simulink model "CDF_VSVPWM_Grid_Parallel"  (R2024b)
settings: Solver=VariableStepAuto  StartTime=0.0  StopTime=0.1  FixedStep=1e-7  MaxStep=1e-5  RelTol=1e-4
InitFcn:
  | Ts=1/10e3;
  | Udc=600;
  | C=4000e-6;
  | m=0.7;
libraries: simulink x24, spsMosfetLib x24, spsSeriesRLCBranchLib x16, spsDiodeLib x12, spsabctoAlphaBetaZeroLib x5, spsThreePhaseVIMeasurementLib x4, spsVoltageMeasurementLib x4, spsAlphaBetaZerotoabcLib x2, spsStairGeneratorLib x2, spsOnDelayLib x2, spsdq0toAlphaBetaZeroLib x2, spsBreakerLib x1
(part 2 of 5)

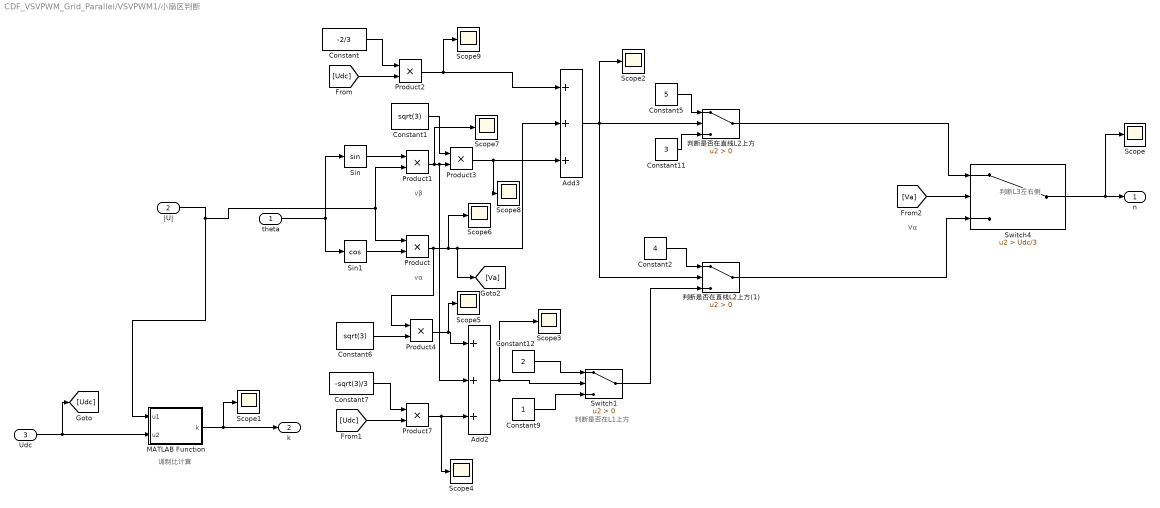
== system CDF_VSVPWM_Grid_Parallel/VSVPWM1/小扇区判断 ==
  note: Vα
  note: 判断L3左右侧
  note: vα
  note: 调制比计算
  note: vβ
  note: 判断是否在L1上方
  theta : Inport
  |U| : Inport  Port=2
  Udc : Inport  Port=3
  "   判断是否在直线L2上方" : Switch  Criteria=u2 > Threshold  SaturateOnIntegerOverflow=off  InputSameDT=off
  "   判断是否在直线L2上方(1)" : Switch  Criteria=u2 > Threshold  SaturateOnIntegerOverflow=off  InputSameDT=off
  Add2 : Sum  Inputs=+++
  Add3 : Sum  Inputs=+++
  Constant : Constant  Value=-2/3
  Constant1 : Constant  Value=sqrt(3)
  Constant11 : Constant  Value=3
  Constant12 : Constant  Value=2
  Constant2 : Constant  Value=4
  Constant5 : Constant  Value=5
  Constant6 : Constant  Value=sqrt(3)
  Constant7 : Constant  Value=-sqrt(3)/3
  Constant9 : Constant
  MATLAB Function : MATLAB Function  TreatAsAtomicUnit=on
  Product{,1..4,7} (6 alike) : Product
  Scope{,1..9} (10 alike) : Scope
  Sin : Trigonometry
  Sin1 : Trigonometry  Operator=cos
  Switch1 : Switch  Criteria=u2 > Threshold  SaturateOnIntegerOverflow=off  InputSameDT=off
  Switch4 : Switch  Criteria=u2 > Threshold  Threshold=Udc/3  SaturateOnIntegerOverflow=off  InputSameDT=off
  n : Outport
  k : Outport  Port=2
  wires:
  Udc/1 -> [Udc], MATLAB Function/2(u2)
  |U|/1 -> MATLAB Function/1(u1), Product1/2, Product/1
  MATLAB Function/1(k) -> Scope1/1, k/1
  theta/1 -> Sin1/1, Sin/1
  Constant/1 -> Product2/1
  [Udc] -> Product2/2
  Constant5/1 -> "   判断是否在直线L2上方"/1
  Switch4/1 -> Scope/1, n/1
  Sin/1 -> Product1/1
  Sin1/1 -> Product/2
  Constant11/1 -> "   判断是否在直线L2上方"/3
  Product1/1 -> Add2/2, Product3/2, Scope7/1
  Constant1/1 -> Product3/1
  Product3/1 -> Scope8/1, Add3/3
  Product/1 -> Scope6/1, [Va], Add3/2, Product4/1
  Product2/1 -> Scope9/1, Add3/1
  Add3/1 -> "   判断是否在直线L2上方(1)"/2, Scope2/1, "   判断是否在直线L2上方"/2
  "   判断是否在直线L2上方"/1 -> Switch4/1
  Constant2/1 -> "   判断是否在直线L2上方(1)"/1
  Constant12/1 -> Switch1/1
  Constant9/1 -> Switch1/3
  Constant6/1 -> Product4/2
  Product4/1 -> Scope5/1, Add2/1
  [Udc] -> Product7/2
  Constant7/1 -> Product7/1
  Product7/1 -> Scope4/1, Add2/3
  Add2/1 -> Scope3/1, Switch1/2
  Switch1/1 -> "   判断是否在直线L2上方(1)"/3
  "   判断是否在直线L2上方(1)"/1 -> Switch4/3
  [Va] -> Switch4/2

== system CDF_VSVPWM_Grid_Parallel/VSVPWM1/生成时间调制波 ==
  note: 调制信号生成
  note: 小扇区
  note: 大扇区
  N : Inport
  n : Inport  Port=2
  T1 : Inport  Port=3
  T2 : Inport  Port=4
  T3 : Inport  Port=5
  T4 : Inport  Port=6
  T5 : Inport  Port=7
  Demux : Demux  Outputs=6
  Gain : Gain  Gain=1/Ts
  Multiport Switch : MultiPortSwitch  Inputs=6  SaturateOnIntegerOverflow=off  InputSameDT=off
  Mux : Mux  Inputs=5
  Scope10 : Scope  NumInputPorts=6
  Scope11 : Scope  NumInputPorts=3
  Scope12 : Scope  NumInputPorts=3
  Scope13 : Scope  NumInputPorts=3
  Scope6 : Scope
  Scope9 : Scope  NumInputPorts=2
  Ⅰ扇区的调制信号计算 : Subsystem
  Ⅱ扇区的调制信号计算 : Subsystem
  Ⅲ扇区的调制信号计算1 : Subsystem
  Ⅳ扇区的调制信号计算 : Subsystem
  Ⅴ扇区的调制信号计算 : Subsystem
  Ⅵ扇区的调制信号计算 : Subsystem
  Tabc12 : Outport
  wires:
  T2/1 -> Mux/2
  T3/1 -> Mux/3
  T1/1 -> Mux/1
  Mux/1 -> Ⅳ扇区的调制信号计算/2(T12345), Ⅵ扇区的调制信号计算/2(T12345), Ⅴ扇区的调制信号计算/2(T12345), Ⅲ扇区的调制信号计算1/2(T12345), Ⅱ扇区的调制信号计算/2(T12345), Ⅰ扇区的调制信号计算/2(T12345)
  n/1 -> Ⅵ扇区的调制信号计算/1(n), Ⅴ扇区的调制信号计算/1(n), Ⅳ扇区的调制信号计算/1(n), Ⅲ扇区的调制信号计算1/1(n), Ⅱ扇区的调制信号计算/1(n), Ⅰ扇区的调制信号计算/1(n)
  Ⅰ扇区的调制信号计算/1(Tabc12) -> Scope12/2, Multiport Switch/2
  Ⅱ扇区的调制信号计算/1(Tabc12) -> Scope11/2, Multiport Switch/3
  N/1 -> Scope13/2, Scope11/1, Scope12/1, Scope9/2, Multiport Switch/1
  Ⅲ扇区的调制信号计算1/1(Tabc12) -> Scope13/1, Multiport Switch/4
  Ⅴ扇区的调制信号计算/1(Tabc12) -> Multiport Switch/6
  Ⅳ扇区的调制信号计算/1(Tabc12) -> Multiport Switch/5
  Ⅵ扇区的调制信号计算/1(Tabc12) -> Scope6/1, Multiport Switch/7
  T4/1 -> Mux/4
  T5/1 -> Mux/5
  ? -> ?
  Gain/1 -> Scope9/1, Tabc12/1
  Multiport Switch/1 -> Scope13/3, Scope11/3, Scope12/3, Demux/1, Gain/1
  Demux/1 -> Scope10/1
  Demux/2 -> Scope10/2
  Demux/3 -> Scope10/3
  Demux/4 -> Scope10/4
  Demux/5 -> Scope10/5
  Demux/6 -> Scope10/6

== system CDF_VSVPWM_Grid_Parallel/VSVPWM1/矢量作用时间计算 ==
  note: 矢量作用时长
  note: 小扇区
  note: 目标矢量角度
  note: 采样时间
  note: 调制比
  n : Inport
  k : Inport  Port=2
  theta : Inport  Port=3
  Ts : Inport  Port=4
  Demux : Demux  Outputs=3
  I1 : Subsystem
  I2 : Subsystem
  I3 : Subsystem
  I4 : Subsystem
  I5 : Subsystem
  Multiport Switch : MultiPortSwitch  Inputs=5  SaturateOnIntegerOverflow=off  InputSameDT=off
  Scope : Scope  NumInputPorts=3
  Ta : Outport
  Tb : Outport  Port=2
  Tc : Outport  Port=3
  wires:
  theta/1 -> I2/3(theta), I3/3(theta), I5/3(theta), I4/3(theta), I1/3(theta)
  Ts/1 -> I2/2(Ts), I3/2(Ts), I5/2(Ts), I4/2(Ts), I1/2(Ts)
  k/1 -> I2/1(k), I3/1(k), I5/1(k), I4/1(k), I1/1(k)
  n/1 -> Multiport Switch/1
  Multiport Switch/1 -> Demux/1
  I1/1(T) -> Multiport Switch/2
  I2/1(T) -> Multiport Switch/3
  I3/1(T) -> Multiport Switch/4
  I4/1(T) -> Multiport Switch/5
  I5/1(T) -> Multiport Switch/6
  Demux/1 -> Scope/1, Ta/1
  Demux/2 -> Scope/2, Tb/1
  Demux/3 -> Scope/3, Tc/1

== system CDF_VSVPWM_Grid_Parallel/VSVPWM/生成时间调制波/Ⅰ扇区的调制信号计算 ==
  n : Inport
  T12345 : Inport  Port=2
  1-1 : Subsystem
  1-2 : Subsystem
  1-3 : Subsystem
  1-4 : Subsystem
  1-5 : Subsystem
  Multiport Switch : MultiPortSwitch  Inputs=5  DiagnosticForDefault=None  SaturateOnIntegerOverflow=off  InputSameDT=off
  Scope : Scope  NumInputPorts=7
  Tabc12 : Outport
  wires:
  n/1 -> Scope/6, Multiport Switch/1
  T12345/1 -> 1-3/1(time), 1-5/1(time), 1-4/1(time), 1-2/1(time), 1-1/1(time)
  1-1/1(Tabc12) -> Scope/1, Multiport Switch/2
  1-2/1(Tabc12) -> Scope/2, Multiport Switch/3
  1-3/1(Tabc12) -> Scope/3, Multiport Switch/4
  1-4/1(Tabc12) -> Scope/4, Multiport Switch/5
  1-5/1(Tabc12) -> Scope/5, Multiport Switch/6
  Multiport Switch/1 -> Scope/7, Tabc12/1

== system CDF_VSVPWM_Grid_Parallel/VSVPWM/生成时间调制波/Ⅱ扇区的调制信号计算 ==
  n : Inport
  T12345 : Inport  Port=2
  2-1 : Subsystem
  2-2 : Subsystem
  2-3 : Subsystem
  2-4 : Subsystem
  2-5 : Subsystem
  Multiport Switch : MultiPortSwitch  Inputs=5  DiagnosticForDefault=None  SaturateOnIntegerOverflow=off  InputSameDT=off
  Scope : Scope  NumInputPorts=7
  Tabc12 : Outport
  wires:
  Multiport Switch/1 -> Scope/7, Tabc12/1
  2-5/1(Tabc12) -> Scope/5, Multiport Switch/6
  2-4/1(Tabc12) -> Scope/4, Multiport Switch/5
  2-3/1(Tabc12) -> Multiport Switch/4, Scope/3
  2-2/1(Tabc12) -> Multiport Switch/3, Scope/2
  2-1/1(Tabc12) -> Scope/1, Multiport Switch/2
  T12345/1 -> 2-2/1(time), 2-1/1(time), 2-3/1(time), 2-5/1(time), 2-4/1(time)
  n/1 -> Multiport Switch/1, Scope/6

== system CDF_VSVPWM_Grid_Parallel/VSVPWM/生成时间调制波/Ⅲ扇区的调制信号计算1 ==
  n : Inport
  T12345 : Inport  Port=2
  1-1 : Subsystem
  1-2 : Subsystem
  1-3 : Subsystem
  1-4 : Subsystem
  1-5 : Subsystem
  Multiport Switch : MultiPortSwitch  Inputs=5  DiagnosticForDefault=None  SaturateOnIntegerOverflow=off  InputSameDT=off
  Scope : Scope  NumInputPorts=7
  Scope1 : Scope
  Tabc12 : Outport
  wires:
  Multiport Switch/1 -> Scope1/1, Scope/7, Tabc12/1
  1-5/1(Tabc12) -> Scope/5, Multiport Switch/6
  1-4/1(Tabc12) -> Scope/4, Multiport Switch/5
  1-3/1(Tabc12) -> Scope/3, Multiport Switch/4
  1-2/1(Tabc12) -> Scope/2, Multiport Switch/3
  1-1/1(Tabc12) -> Scope/1, Multiport Switch/2
  T12345/1 -> 1-1/1(time), 1-2/1(time), 1-4/1(time), 1-5/1(time), 1-3/1(time)
  n/1 -> Multiport Switch/1, Scope/6

== system CDF_VSVPWM_Grid_Parallel/VSVPWM/生成时间调制波/Ⅳ扇区的调制信号计算 ==
  n : Inport
  T12345 : Inport  Port=2
  2-1 : Subsystem
  2-2 : Subsystem
  2-3 : Subsystem
  2-4 : Subsystem
  2-5 : Subsystem
  Multiport Switch : MultiPortSwitch  Inputs=5  DiagnosticForDefault=None  SaturateOnIntegerOverflow=off  InputSameDT=off
  Scope : Scope  NumInputPorts=7
  Scope1 : Scope
  Tabc12 : Outport
  wires:
  n/1 -> Scope/6, Multiport Switch/1
  T12345/1 -> 2-4/1(time), 2-5/1(time), 2-3/1(time), 2-1/1(time), 2-2/1(time)
  2-1/1(Tabc12) -> Scope/1, Multiport Switch/2
  2-2/1(Tabc12) -> Scope/2, Multiport Switch/3
  2-3/1(Tabc12) -> Scope/3, Multiport Switch/4
  2-4/1(Tabc12) -> Scope/4, Multiport Switch/5
  2-5/1(Tabc12) -> Scope/5, Multiport Switch/6
  Multiport Switch/1 -> Scope1/1, Scope/7, Tabc12/1

== system CDF_VSVPWM_Grid_Parallel/VSVPWM/生成时间调制波/Ⅴ扇区的调制信号计算 ==
  n : Inport
  T12345 : Inport  Port=2
  1-1 : Subsystem
  1-2 : Subsystem
  1-3 : Subsystem
  1-4 : Subsystem
  1-5 : Subsystem
  Multiport Switch : MultiPortSwitch  Inputs=5  DiagnosticForDefault=None  SaturateOnIntegerOverflow=off  InputSameDT=off
  Scope : Scope  NumInputPorts=7
  Scope1 : Scope
  Tabc12 : Outport
  wires:
  Multiport Switch/1 -> Scope1/1, Scope/7, Tabc12/1
  1-5/1(Tabc12) -> Scope/5, Multiport Switch/6
  1-4/1(Tabc12) -> Scope/4, Multiport Switch/5
  1-3/1(Tabc12) -> Scope/3, Multiport Switch/4
  1-2/1(Tabc12) -> Multiport Switch/3, Scope/2
  1-1/1(Tabc12) -> Scope/1, Multiport Switch/2
  T12345/1 -> 1-1/1(time), 1-2/1(time), 1-4/1(time), 1-5/1(time), 1-3/1(time)
  n/1 -> Scope/6, Multiport Switch/1

== system CDF_VSVPWM_Grid_Parallel/VSVPWM/生成时间调制波/Ⅵ扇区的调制信号计算 ==
  n : Inport
  T12345 : Inport  Port=2
  2-1 : Subsystem
  2-2 : Subsystem
  2-3 : Subsystem
  2-4 : Subsystem
  2-5 : Subsystem
  Multiport Switch : MultiPortSwitch  Inputs=5  DiagnosticForDefault=None  SaturateOnIntegerOverflow=off  InputSameDT=off
  Tabc12 : Outport
  wires:
  n/1 -> Multiport Switch/1
  T12345/1 -> 2-4/1(time), 2-5/1(time), 2-3/1(time), 2-1/1(time), 2-2/1(time)
  2-1/1(Tabc12) -> Multiport Switch/2
  2-2/1(Tabc12) -> Multiport Switch/3
  2-3/1(Tabc12) -> Multiport Switch/4
  2-4/1(Tabc12) -> Multiport Switch/5
  2-5/1(Tabc12) -> Multiport Switch/6
  Multiport Switch/1 -> Tabc12/1

== system CDF_VSVPWM_Grid_Parallel/VSVPWM/矢量作用时间计算/I1 ==
  note: Ta
  note: Tb
  note: Tc
  k : Inport
  Ts : Inport  Port=2
  theta : Inport  Port=3
  Constant : Constant
  Gain : Gain  Gain=2
  Mux : Mux  Inputs=3
  Product : Product  Inputs=3
  Product1 : Product  Inputs=3
  Product2 : Product
  Product3 : Product
  Scope : Scope
  Sin : Trigonometry
  Sum : Sum  Inputs=|+-
  sin(pi/3+u) : MATLAB Function  TreatAsAtomicUnit=on
  sin(pi/3-u) : MATLAB Function  TreatAsAtomicUnit=on
  T : Outport
  wires:
  k/1 -> Gain/1
  theta/1 -> sin(pi/3-u)/1(u), sin(pi/3+u)/1(u), Sin/1
  Ts/1 -> Product/2, Product2/2, Product1/2
  Product1/1 -> Mux/2
  Product/1 -> Mux/1
  Mux/1 -> Scope/1, T/1
  sin(pi/3-u)/1(y) -> Product/3
  Gain/1 -> Product3/1, Product1/1, Product/1
  Sin/1 -> Product1/3
  Product2/1 -> Mux/3
  sin(pi/3+u)/1(y) -> Product3/2
  Product3/1 -> Sum/2
  Constant/1 -> Sum/1
  Sum/1 -> Product2/1

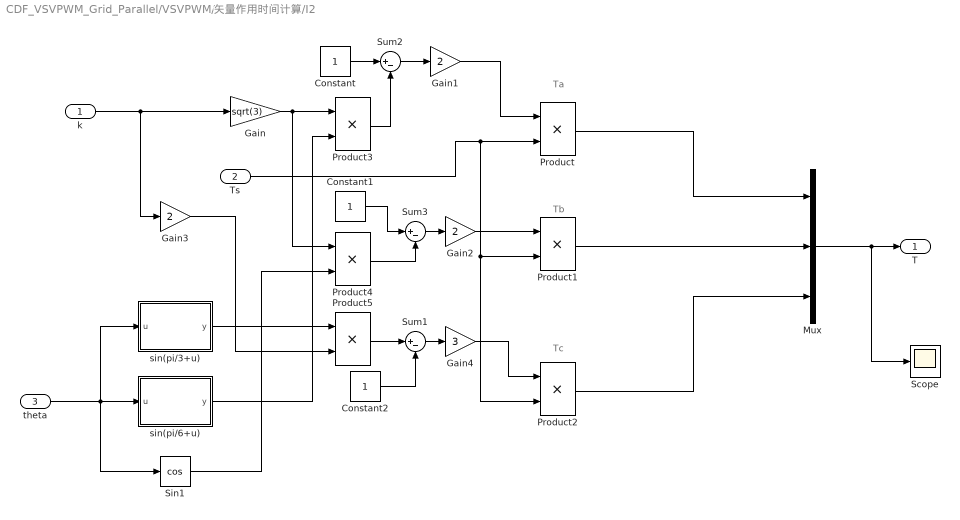
== system CDF_VSVPWM_Grid_Parallel/VSVPWM/矢量作用时间计算/I2 ==
  note: Tc
  note: Tb
  note: Ta
  k : Inport
  Ts : Inport  Port=2
  theta : Inport  Port=3
  Constant : Constant
  Constant1 : Constant
  Constant2 : Constant
  Gain : Gain  Gain=sqrt(3)
  Gain1 : Gain  Gain=2
  Gain2 : Gain  Gain=2
  Gain3 : Gain  Gain=2
  Gain4 : Gain  Gain=3
  Mux : Mux  Inputs=3
  Product{,1..5} (6 alike) : Product
  Scope : Scope
  Sin1 : Trigonometry  Operator=cos
  Sum1 : Sum  Inputs=|+-
  Sum2 : Sum  Inputs=|+-
  Sum3 : Sum  Inputs=|+-
  sin(pi/3+u) : MATLAB Function  TreatAsAtomicUnit=on
  sin(pi/6+u) : MATLAB Function  TreatAsAtomicUnit=on
  T : Outport
  wires:
  Product2/1 -> Mux/3
  Product/1 -> Mux/1
  Product1/1 -> Mux/2
  theta/1 -> Sin1/1, sin(pi/6+u)/1(u), sin(pi/3+u)/1(u)
  k/1 -> Gain3/1, Gain/1
  Gain/1 -> Product4/1, Product3/1
  sin(pi/6+u)/1(y) -> Product3/2
  Product3/1 -> Sum2/2
  Constant/1 -> Sum2/1
  Sum2/1 -> Gain1/1
  Gain1/1 -> Product/1
  Ts/1 -> Product2/2, Product1/2, Product/2
  Sin1/1 -> Product4/2
  Product4/1 -> Sum3/2
  Constant1/1 -> Sum3/1
  Sum3/1 -> Gain2/1
  Gain2/1 -> Product1/1
  sin(pi/3+u)/1(y) -> Product5/1
  Gain3/1 -> Product5/2
  Product5/1 -> Sum1/1
  Constant2/1 -> Sum1/2
  Sum1/1 -> Gain4/1
  Gain4/1 -> Product2/1
  Mux/1 -> Scope/1, T/1

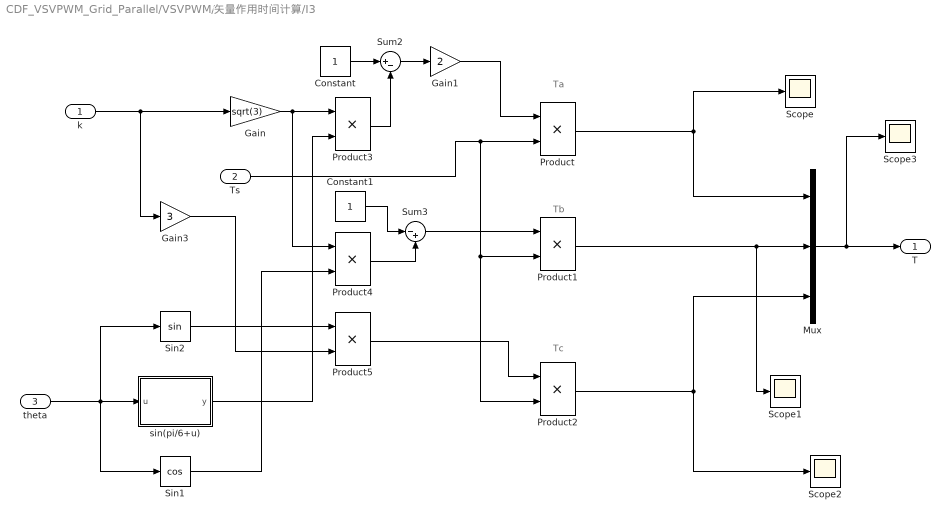
== system CDF_VSVPWM_Grid_Parallel/VSVPWM/矢量作用时间计算/I3 ==
  note: Ta
  note: Tb
  note: Tc
  k : Inport
  Ts : Inport  Port=2
  theta : Inport  Port=3
  Constant : Constant
  Constant1 : Constant
  Gain : Gain  Gain=sqrt(3)
  Gain1 : Gain  Gain=2
  Gain3 : Gain  Gain=3
  Mux : Mux  Inputs=3
  Product{,1..5} (6 alike) : Product
  Scope{,1..3} (4 alike) : Scope
  Sin1 : Trigonometry  Operator=cos
  Sin2 : Trigonometry
  Sum2 : Sum  Inputs=|+-
  Sum3 : Sum  Inputs=|-+
  sin(pi/6+u) : MATLAB Function  TreatAsAtomicUnit=on
  T : Outport
  wires:
  Product5/1 -> Product2/1
  Gain3/1 -> Product5/2
  Sin2/1 -> Product5/1
  Sum3/1 -> Product1/1
  Constant1/1 -> Sum3/1
  Product4/1 -> Sum3/2
  Sin1/1 -> Product4/2
  Ts/1 -> Product/2, Product1/2, Product2/2
  Gain1/1 -> Product/1
  Sum2/1 -> Gain1/1
  Constant/1 -> Sum2/1
  Product3/1 -> Sum2/2
  sin(pi/6+u)/1(y) -> Product3/2
  Gain/1 -> Product3/1, Product4/1
  k/1 -> Gain/1, Gain3/1
  theta/1 -> Sin2/1, sin(pi/6+u)/1(u), Sin1/1
  Product1/1 -> Scope1/1, Mux/2
  Product/1 -> Scope/1, Mux/1
  Mux/1 -> Scope3/1, T/1
  Product2/1 -> Mux/3, Scope2/1

== system CDF_VSVPWM_Grid_Parallel/VSVPWM/矢量作用时间计算/I4 ==
  note: Ta
  note: Tb
  note: Tc
  k : Inport
  Ts : Inport  Port=2
  theta : Inport  Port=3
  Constant : Constant
  Constant1 : Constant
  Gain : Gain  Gain=sqrt(3)
  Gain1 : Gain  Gain=2
  Gain3 : Gain  Gain=3
  Mux : Mux  Inputs=3
  Product{,1..5} (6 alike) : Product
  Scope{,1..3} (4 alike) : Scope
  Sin1 : Trigonometry  Operator=cos
  Sum2 : Sum  Inputs=|+-
  Sum3 : Sum  Inputs=|+-
  sin(pi/3-u) : MATLAB Function  TreatAsAtomicUnit=on
  sin(pi/6+u) : MATLAB Function  TreatAsAtomicUnit=on
  T : Outport
  wires:
  Sum3/1 -> Product1/1
  Gain1/1 -> Product/1
  Sum2/1 -> Gain1/1
  Constant/1 -> Sum2/1
  Product3/1 -> Sum2/2
  Gain/1 -> Product4/1, Product3/1
  k/1 -> Gain/1, Gain3/1
  theta/1 -> sin(pi/3-u)/1(u), sin(pi/6+u)/1(u), Sin1/1
  Product1/1 -> Scope2/1, Mux/2
  Product/1 -> Scope1/1, Mux/1
  Mux/1 -> Scope/1, T/1
  Product2/1 -> Mux/3, Scope3/1
  Sin1/1 -> Product3/2
  Ts/1 -> Product2/2, Product1/2, Product/2
  sin(pi/6+u)/1(y) -> Product4/2
  Constant1/1 -> Sum3/2
  Product4/1 -> Sum3/1
  sin(pi/3-u)/1(y) -> Product5/2
  Gain3/1 -> Product5/1
  Product5/1 -> Product2/1

== system CDF_VSVPWM_Grid_Parallel/VSVPWM/矢量作用时间计算/I5 ==
  note: Tc
  note: Tb
  note: Ta
  k : Inport
  Ts : Inport  Port=2
  theta : Inport  Port=3
  Constant : Constant
  Constant1 : Constant
  Gain : Gain  Gain=sqrt(3)
  Gain1 : Gain  Gain=3
  Mux : Mux  Inputs=3
  Product{,1..5} (6 alike) : Product
  Scope : Scope
  Scope1 : Scope
  Scope2 : Scope
  Sin1 : Trigonometry  Operator=cos
  Sum1 : Sum  Inputs=|+-
  Sum2 : Sum  Inputs=|-+
  Sum3 : Sum  Inputs=|+-
  sin(pi/3+u) : MATLAB Function  TreatAsAtomicUnit=on
  sin(pi/6+u) : MATLAB Function  TreatAsAtomicUnit=on
  T : Outport
  wires:
  k/1 -> Product5/1, Gain/1
  sin(pi/3+u)/1(y) -> Product5/2
  Product4/1 -> Sum3/1
  Constant1/1 -> Sum1/1, Sum3/2
  sin(pi/6+u)/1(y) -> Product4/2
  Ts/1 -> Product/2, Product1/2, Product2/2
  Sin1/1 -> Product3/2
  Mux/1 -> T/1
  Product/1 -> Mux/1, Scope/1
  Product1/1 -> Scope1/1, Mux/2
  theta/1 -> Sin1/1, sin(pi/6+u)/1(u), sin(pi/3+u)/1(u)
  Gain/1 -> Product3/1, Product4/1
  Product3/1 -> Sum2/2
  Constant/1 -> Sum2/1
  Sum2/1 -> Product/1
  Sum3/1 -> Product1/1
  Product5/1 -> Sum1/2
  Sum1/1 -> Product2/1
  Product2/1 -> Gain1/1
  Gain1/1 -> Mux/3, Scope2/1

== system CDF_VSVPWM_Grid_Parallel/VSVPWM1/生成时间调制波/Ⅰ扇区的调制信号计算 ==
  n : Inport
  T12345 : Inport  Port=2
  1-1 : Subsystem
  1-2 : Subsystem
  1-3 : Subsystem
  1-4 : Subsystem
  1-5 : Subsystem
  Multiport Switch : MultiPortSwitch  Inputs=5  DiagnosticForDefault=None  SaturateOnIntegerOverflow=off  InputSameDT=off
  Scope : Scope  NumInputPorts=7
  Tabc12 : Outport
  wires:
  n/1 -> Scope/6, Multiport Switch/1
  T12345/1 -> 1-3/1(time), 1-5/1(time), 1-4/1(time), 1-2/1(time), 1-1/1(time)
  1-1/1(Tabc12) -> Scope/1, Multiport Switch/2
  1-2/1(Tabc12) -> Scope/2, Multiport Switch/3
  1-3/1(Tabc12) -> Scope/3, Multiport Switch/4
  1-4/1(Tabc12) -> Scope/4, Multiport Switch/5
  1-5/1(Tabc12) -> Scope/5, Multiport Switch/6
  Multiport Switch/1 -> Scope/7, Tabc12/1

== system CDF_VSVPWM_Grid_Parallel/VSVPWM1/生成时间调制波/Ⅱ扇区的调制信号计算 ==
  n : Inport
  T12345 : Inport  Port=2
  2-1 : Subsystem
  2-2 : Subsystem
  2-3 : Subsystem
  2-4 : Subsystem
  2-5 : Subsystem
  Multiport Switch : MultiPortSwitch  Inputs=5  DiagnosticForDefault=None  SaturateOnIntegerOverflow=off  InputSameDT=off
  Scope : Scope  NumInputPorts=7
  Tabc12 : Outport
  wires:
  Multiport Switch/1 -> Scope/7, Tabc12/1
  2-5/1(Tabc12) -> Scope/5, Multiport Switch/6
  2-4/1(Tabc12) -> Scope/4, Multiport Switch/5
  2-3/1(Tabc12) -> Multiport Switch/4, Scope/3
  2-2/1(Tabc12) -> Multiport Switch/3, Scope/2
  2-1/1(Tabc12) -> Scope/1, Multiport Switch/2
  T12345/1 -> 2-2/1(time), 2-1/1(time), 2-3/1(time), 2-5/1(time), 2-4/1(time)
  n/1 -> Multiport Switch/1, Scope/6

== system CDF_VSVPWM_Grid_Parallel/VSVPWM1/生成时间调制波/Ⅲ扇区的调制信号计算1 ==
  n : Inport
  T12345 : Inport  Port=2
  1-1 : Subsystem
  1-2 : Subsystem
  1-3 : Subsystem
  1-4 : Subsystem
  1-5 : Subsystem
  Multiport Switch : MultiPortSwitch  Inputs=5  DiagnosticForDefault=None  SaturateOnIntegerOverflow=off  InputSameDT=off
  Scope : Scope  NumInputPorts=7
  Scope1 : Scope
  Tabc12 : Outport
  wires:
  Multiport Switch/1 -> Scope1/1, Scope/7, Tabc12/1
  1-5/1(Tabc12) -> Scope/5, Multiport Switch/6
  1-4/1(Tabc12) -> Scope/4, Multiport Switch/5
  1-3/1(Tabc12) -> Scope/3, Multiport Switch/4
  1-2/1(Tabc12) -> Scope/2, Multiport Switch/3
  1-1/1(Tabc12) -> Scope/1, Multiport Switch/2
  T12345/1 -> 1-1/1(time), 1-2/1(time), 1-4/1(time), 1-5/1(time), 1-3/1(time)
  n/1 -> Multiport Switch/1, Scope/6

== system CDF_VSVPWM_Grid_Parallel/VSVPWM1/生成时间调制波/Ⅳ扇区的调制信号计算 ==
  n : Inport
  T12345 : Inport  Port=2
  2-1 : Subsystem
  2-2 : Subsystem
  2-3 : Subsystem
  2-4 : Subsystem
  2-5 : Subsystem
  Multiport Switch : MultiPortSwitch  Inputs=5  DiagnosticForDefault=None  SaturateOnIntegerOverflow=off  InputSameDT=off
  Scope : Scope  NumInputPorts=7
  Scope1 : Scope
  Tabc12 : Outport
  wires:
  n/1 -> Scope/6, Multiport Switch/1
  T12345/1 -> 2-4/1(time), 2-5/1(time), 2-3/1(time), 2-1/1(time), 2-2/1(time)
  2-1/1(Tabc12) -> Scope/1, Multiport Switch/2
  2-2/1(Tabc12) -> Scope/2, Multiport Switch/3
  2-3/1(Tabc12) -> Scope/3, Multiport Switch/4
  2-4/1(Tabc12) -> Scope/4, Multiport Switch/5
  2-5/1(Tabc12) -> Scope/5, Multiport Switch/6
  Multiport Switch/1 -> Scope1/1, Scope/7, Tabc12/1

== system CDF_VSVPWM_Grid_Parallel/VSVPWM1/生成时间调制波/Ⅴ扇区的调制信号计算 ==
  n : Inport
  T12345 : Inport  Port=2
  1-1 : Subsystem
  1-2 : Subsystem
  1-3 : Subsystem
  1-4 : Subsystem
  1-5 : Subsystem
  Multiport Switch : MultiPortSwitch  Inputs=5  DiagnosticForDefault=None  SaturateOnIntegerOverflow=off  InputSameDT=off
  Scope : Scope  NumInputPorts=7
  Scope1 : Scope
  Tabc12 : Outport
  wires:
  Multiport Switch/1 -> Scope1/1, Scope/7, Tabc12/1
  1-5/1(Tabc12) -> Scope/5, Multiport Switch/6
  1-4/1(Tabc12) -> Scope/4, Multiport Switch/5
  1-3/1(Tabc12) -> Scope/3, Multiport Switch/4
  1-2/1(Tabc12) -> Multiport Switch/3, Scope/2
  1-1/1(Tabc12) -> Scope/1, Multiport Switch/2
  T12345/1 -> 1-1/1(time), 1-2/1(time), 1-4/1(time), 1-5/1(time), 1-3/1(time)
  n/1 -> Scope/6, Multiport Switch/1

== system CDF_VSVPWM_Grid_Parallel/VSVPWM1/生成时间调制波/Ⅵ扇区的调制信号计算 ==
  n : Inport
  T12345 : Inport  Port=2
  2-1 : Subsystem
  2-2 : Subsystem
  2-3 : Subsystem
  2-4 : Subsystem
  2-5 : Subsystem
  Multiport Switch : MultiPortSwitch  Inputs=5  DiagnosticForDefault=None  SaturateOnIntegerOverflow=off  InputSameDT=off
  Tabc12 : Outport
  wires:
  n/1 -> Multiport Switch/1
  T12345/1 -> 2-4/1(time), 2-5/1(time), 2-3/1(time), 2-1/1(time), 2-2/1(time)
  2-1/1(Tabc12) -> Multiport Switch/2
  2-2/1(Tabc12) -> Multiport Switch/3
  2-3/1(Tabc12) -> Multiport Switch/4
  2-4/1(Tabc12) -> Multiport Switch/5
  2-5/1(Tabc12) -> Multiport Switch/6
  Multiport Switch/1 -> Tabc12/1

== system CDF_VSVPWM_Grid_Parallel/VSVPWM1/矢量作用时间计算/I1 ==
  note: Ta
  note: Tb
  note: Tc
  k : Inport
  Ts : Inport  Port=2
  theta : Inport  Port=3
  Constant : Constant
  Gain : Gain  Gain=2
  Mux : Mux  Inputs=3
  Product : Product  Inputs=3
  Product1 : Product  Inputs=3
  Product2 : Product
  Product3 : Product
  Scope : Scope
  Sin : Trigonometry
  Sum : Sum  Inputs=|+-
  sin(pi/3+u) : MATLAB Function  TreatAsAtomicUnit=on
  sin(pi/3-u) : MATLAB Function  TreatAsAtomicUnit=on
  T : Outport
  wires:
  k/1 -> Gain/1
  theta/1 -> sin(pi/3-u)/1(u), sin(pi/3+u)/1(u), Sin/1
  Ts/1 -> Product/2, Product2/2, Product1/2
  Product1/1 -> Mux/2
  Product/1 -> Mux/1
  Mux/1 -> Scope/1, T/1
  sin(pi/3-u)/1(y) -> Product/3
  Gain/1 -> Product3/1, Product1/1, Product/1
  Sin/1 -> Product1/3
  Product2/1 -> Mux/3
  sin(pi/3+u)/1(y) -> Product3/2
  Product3/1 -> Sum/2
  Constant/1 -> Sum/1
  Sum/1 -> Product2/1

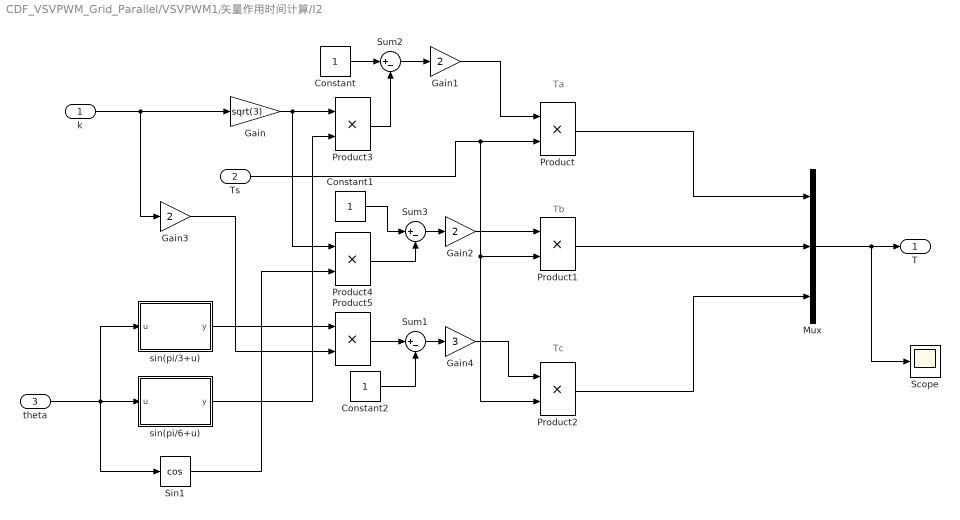
== system CDF_VSVPWM_Grid_Parallel/VSVPWM1/矢量作用时间计算/I2 ==
  note: Tc
  note: Tb
  note: Ta
  k : Inport
  Ts : Inport  Port=2
  theta : Inport  Port=3
  Constant : Constant
  Constant1 : Constant
  Constant2 : Constant
  Gain : Gain  Gain=sqrt(3)
  Gain1 : Gain  Gain=2
  Gain2 : Gain  Gain=2
  Gain3 : Gain  Gain=2
  Gain4 : Gain  Gain=3
  Mux : Mux  Inputs=3
  Product{,1..5} (6 alike) : Product
  Scope : Scope
  Sin1 : Trigonometry  Operator=cos
  Sum1 : Sum  Inputs=|+-
  Sum2 : Sum  Inputs=|+-
  Sum3 : Sum  Inputs=|+-
  sin(pi/3+u) : MATLAB Function  TreatAsAtomicUnit=on
  sin(pi/6+u) : MATLAB Function  TreatAsAtomicUnit=on
  T : Outport
  wires:
  Product2/1 -> Mux/3
  Product/1 -> Mux/1
  Product1/1 -> Mux/2
  theta/1 -> Sin1/1, sin(pi/6+u)/1(u), sin(pi/3+u)/1(u)
  k/1 -> Gain3/1, Gain/1
  Gain/1 -> Product4/1, Product3/1
  sin(pi/6+u)/1(y) -> Product3/2
  Product3/1 -> Sum2/2
  Constant/1 -> Sum2/1
  Sum2/1 -> Gain1/1
  Gain1/1 -> Product/1
  Ts/1 -> Product2/2, Product1/2, Product/2
  Sin1/1 -> Product4/2
  Product4/1 -> Sum3/2
  Constant1/1 -> Sum3/1
  Sum3/1 -> Gain2/1
  Gain2/1 -> Product1/1
  sin(pi/3+u)/1(y) -> Product5/1
  Gain3/1 -> Product5/2
  Product5/1 -> Sum1/1
  Constant2/1 -> Sum1/2
  Sum1/1 -> Gain4/1
  Gain4/1 -> Product2/1
  Mux/1 -> Scope/1, T/1
